simulink model "ClimaCmftFlowMgr"  (R2022b)
settings: Solver=FixedStepDiscrete  StartTime=0.0  StopTime=10.0  FixedStep=1  RelTol=1e-3
settings Model: Creator=blue.ge  LastModifiedBy=blue.ge
settings DataIOCC: SaveFormat=StructureWithTime
settings OptimizationCC: DefaultParameterBehavior=Inlined  EnhancedBackFolding=on  PassReuseOutputArgsAs=Individual arguments  ZeroExternalMemoryAtStartup=off  ZeroInternalMemoryAtStartup=off  OptimizeBlockOrder=speed  DifferentSizesBufferReuse=on  OptimizationCustomize=off  EfficientTunableParamExpr=on
settings DebuggingCC: ParameterTunabilityLossMsg=error
settings ModelReferenceCC: ModelReferenceNumInstancesAllowed=Single
settings SFSimCC: MATLABDynamicMemAlloc=off
settings RTWCC: Description=AUTOSAR  SystemTargetFile=autosar.tlc  GenCodeOnly=on  TemplateMakefile=ert_default_tmf
settings CodeAppCC: ForceParamTrailComments=on  IgnoreCustomStorageClasses=off  OperatorAnnotations=on  SimulinkDataObjDesc=on  SFDataObjDesc=on  InsertBlockDesc=on
settings STFCustomTargetCC: TargetLangStandard=C99 (ISO)  MultiwordLength=256  ParMdlRefBuildCompliant=on  CompOptLevelCompliant=on  IncludeMdlTerminateFcn=off  CombineOutputUpdateFcns=on  SuppressErrorStatus=on  ERTCustomFileBanners=on  SupportNonFinite=off  SupportComplex=off  SupportContinuousTime=off  SupportNonInlinedSFcns=off  RemoveResetFunc=on  MATLABClassNameForMDSCustomization=Simulink.SoftwareTarget.ERTCustomization  AutosarCompliant=on  UseToolchainInfoCompliant=on  LookupTableObjectStructAxisOrder=2,1,3,4,...  LUTObjectStructOrderEvenSpacing=Size,Table,Breakpoints  SystemTargetFile=autosar.tlc
settings ERTTargetCC: TargetLangStandard=C99 (ISO)  ConcurrentExecutionCompliant=off  IncludeMdlTerminateFcn=off  SuppressErrorStatus=on  SupportNonFinite=off  SupportComplex=off  ModelStepFunctionPrototypeControlCompliant=off  CPPClassGenCompliant=off  AutosarCompliant=on  LookupTableObjectStructAxisOrder=2,1,3,4,...  LUTObjectStructOrderEvenSpacing=Size,Table,Breakpoints  InlinedParameterPlacement=NonHierarchical  ERTCustomFileTemplate=example_file_process.tlc

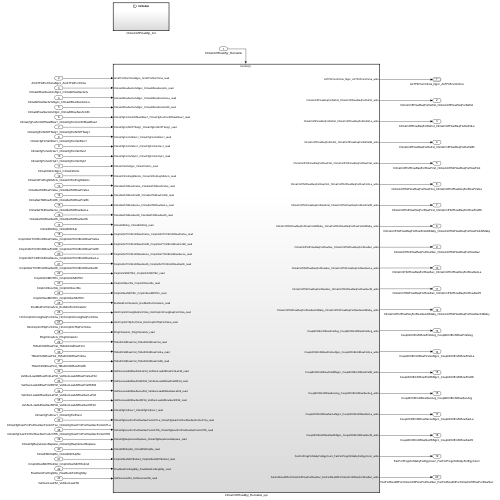
[thumbnail: Simulink's stored preview of the top-level diagram]
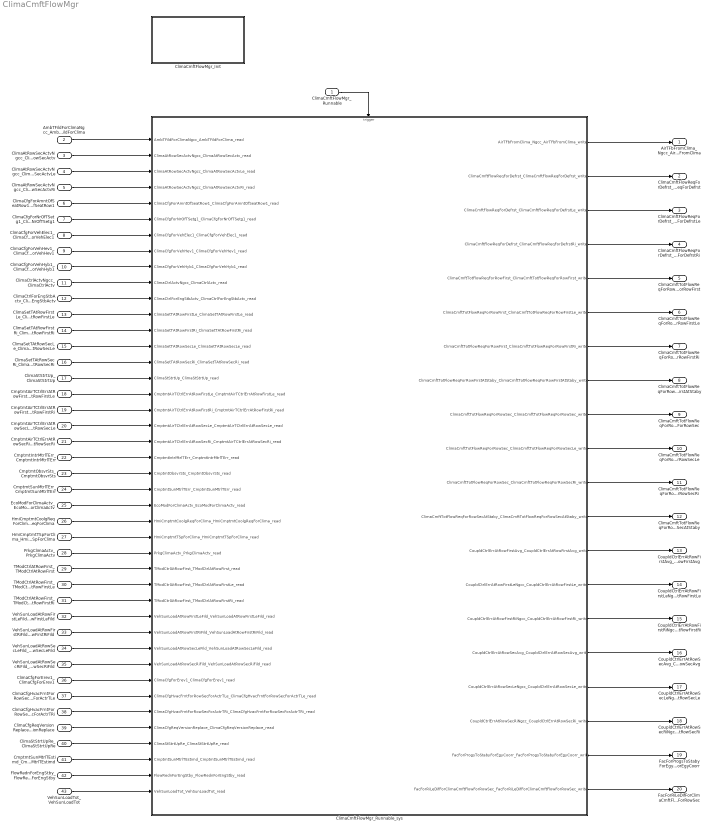
== system ClimaCmftFlowMgr ==
  ClimaCmftFlowMgr_Runnable : Inport  SampleTime=1  OutputFunctionCall=on
  AmbTFildForClimaNgcc_AmbTFildForClima : Inport  Port=2  OutDataTypeStr=T30  PortDimensions=1
  ClimaAtRowSecActvNgcc_ClimaAtRowSecActv : Inport  Port=3  OutDataTypeStr=Boolean  PortDimensions=1
  ClimaAtRowSecActvNgcc_ClimaAtRowSecActvLe : Inport  Port=4  OutDataTypeStr=Boolean  PortDimensions=1
  ClimaAtRowSecActvNgcc_ClimaAtRowSecActvRi : Inport  Port=5  OutDataTypeStr=Boolean  PortDimensions=1
  ClimaCfgForAmntOfSeatRow1_ClimaCfgForAmntOfSeatRow1 : Inport  Port=6  OutDataTypeStr=Enum: ClimaCfgForAmntOfSeatRow1  PortDimensions=1
  ClimaCfgForNrOfTSetg1_ClimaCfgForNrOfTSetg1 : Inport  Port=7  OutDataTypeStr=Enum: ClimaCfgForNrOfTSetg1  PortDimensions=1
  ClimaCfgForVehElec1_ClimaCfgForVehElec1 : Inport  Port=8  OutDataTypeStr=Boolean  PortDimensions=1
  ClimaCfgForVehHev1_ClimaCfgForVehHev1 : Inport  Port=9  OutDataTypeStr=Boolean  PortDimensions=1
  ClimaCfgForVehHyb1_ClimaCfgForVehHyb1 : Inport  Port=10  OutDataTypeStr=Boolean  PortDimensions=1
  ClimaCtrlActvNgcc_ClimaCtrlActv : Inport  Port=11  OutDataTypeStr=Boolean  PortDimensions=1
  ClimaCtrlForEngStbActv_ClimaCtrlForEngStbActv : Inport  Port=12  OutDataTypeStr=Boolean  PortDimensions=1
  ClimaSetTAtRowFirstLe_ClimaSetTAtRowFirstLe : Inport  Port=13  OutDataTypeStr=T30  PortDimensions=1
  ClimaSetTAtRowFirstRi_ClimaSetTAtRowFirstRi : Inport  Port=14  OutDataTypeStr=T30  PortDimensions=1
  ClimaSetTAtRowSecLe_ClimaSetTAtRowSecLe : Inport  Port=15  OutDataTypeStr=T30  PortDimensions=1
  ClimaSetTAtRowSecRi_ClimaSetTAtRowSecRi : Inport  Port=16  OutDataTypeStr=T30  PortDimensions=1
  ClimaStStrtUp_ClimaStStrtUp : Inport  Port=17  OutDataTypeStr=Enum: ClimaStStrtUp  PortDimensions=1
  CmptmtAirTCtrlErrAtRowFirstLe_CmptmtAirTCtrlErrAtRowFirstLe : Inport  Port=18  OutDataTypeStr=T30  PortDimensions=1
  CmptmtAirTCtrlErrAtRowFirstRi_CmptmtAirTCtrlErrAtRowFirstRi : Inport  Port=19  OutDataTypeStr=T30  PortDimensions=1
  CmptmtAirTCtrlErrAtRowSecLe_CmptmtAirTCtrlErrAtRowSecLe : Inport  Port=20  OutDataTypeStr=T30  PortDimensions=1
  CmptmtAirTCtrlErrAtRowSecRi_CmptmtAirTCtrlErrAtRowSecRi : Inport  Port=21  OutDataTypeStr=T30  PortDimensions=1
  CmptmtIntrMtrlTErr_CmptmtIntrMtrlTErr : Inport  Port=22  OutDataTypeStr=T30  PortDimensions=1
  CmptmtObsvrSts_CmptmtObsvrSts : Inport  Port=23  OutDataTypeStr=Enum: ObsvrStsForCmptmt  PortDimensions=1
  CmptmtSunMtrlTErr_CmptmtSunMtrlTErr : Inport  Port=24  OutDataTypeStr=T30  PortDimensions=1
  EcoModForClimaActv_EcoModForClimaActv : Inport  Port=25  OutDataTypeStr=Enum: OnOff1  PortDimensions=1
  HmiCmptmtCoolgReqForClima_HmiCmptmtCoolgReqForClima : Inport  Port=26  OutDataTypeStr=Enum: HmiCmptmtCoolgReq  PortDimensions=1
  HmiCmptmtTSpForClima_HmiCmptmtTSpForClima : Inport  Port=27  OutDataTypeStr=Bus: HmiCmptmtTSp  PortDimensions=1  BusOutputAsStruct=on
  PrkgClimaActv_PrkgClimaActv : Inport  Port=28  OutDataTypeStr=Enum: PrkgClimaActv  PortDimensions=1
  TModCtrlAtRowFirst_TModCtrlAtRowFirst : Inport  Port=29  OutDataTypeStr=T31  PortDimensions=1
  TModCtrlAtRowFirst_TModCtrlAtRowFirstLe : Inport  Port=30  OutDataTypeStr=T31  PortDimensions=1
  TModCtrlAtRowFirst_TModCtrlAtRowFirstRi : Inport  Port=31  OutDataTypeStr=T31  PortDimensions=1
  VehSunLoadAtRowFirstLeFild_VehSunLoadAtRowFirstLeFild : Inport  Port=32  OutDataTypeStr=SunLoad30  PortDimensions=1
  VehSunLoadAtRowFirstRiFild_VehSunLoadAtRowFirstRiFild : Inport  Port=33  OutDataTypeStr=SunLoad30  PortDimensions=1
  VehSunLoadAtRowSecLeFild_VehSunLoadAtRowSecLeFild : Inport  Port=34  OutDataTypeStr=SunLoad30  PortDimensions=1
  VehSunLoadAtRowSecRiFild_VehSunLoadAtRowSecRiFild : Inport  Port=35  OutDataTypeStr=SunLoad30  PortDimensions=1
  ClimaCfgForErev1_ClimaCfgForErev1 : Inport  Port=36  OutDataTypeStr=Boolean  PortDimensions=1
  ClimaCfgHvacFrntForRowSecForActrTLe_ClimaCfgHvacFrntForRowSecForActrTLe : Inport  Port=37  OutDataTypeStr=Boolean  PortDimensions=1
  ClimaCfgHvacFrntForRowSecForActrTRi_ClimaCfgHvacFrntForRowSecForActrTRi : Inport  Port=38  OutDataTypeStr=Boolean  PortDimensions=1
  ClimaCfgReqVersionReplace_ClimaCfgReqVersionReplace : Inport  Port=39  OutDataTypeStr=UInt8  PortDimensions=1
  ClimaStStrtUpRe_ClimaStStrtUpRe : Inport  Port=40  OutDataTypeStr=Enum: ClimaStStrtUp  PortDimensions=1
  CmptmtSunMtrlTEstimd_CmptmtSunMtrlTEstimd : Inport  Port=41  OutDataTypeStr=T30  PortDimensions=1
  FlowRednForEngStby_FlowRednForEngStby : Inport  Port=42  OutDataTypeStr=Boolean  PortDimensions=1
  VehSunLoadTot_VehSunLoadTot : Inport  Port=43  OutDataTypeStr=SunLoad30  PortDimensions=1
  ClimaCmftFlowMgr_Init : Subsystem
  ClimaCmftFlowMgr_Runnable_sys : Subsystem
  AirTFbFromClima_Ngcc_AirTFbFromClima : Outport  OutDataTypeStr=Bus: AirTFbFromClimaRec  PortDimensions=1  BusOutputAsStruct=on
  ClimaCmftFlowReqForDefrst_ClimaCmftFlowReqForDefrst : Outport  Port=2  OutDataTypeStr=AirFlow30  PortDimensions=1
  ClimaCmftFlowReqForDefrst_ClimaCmftFlowReqForDefrstLe : Outport  Port=3  OutDataTypeStr=AirFlow30  PortDimensions=1
  ClimaCmftFlowReqForDefrst_ClimaCmftFlowReqForDefrstRi : Outport  Port=4  OutDataTypeStr=AirFlow30  PortDimensions=1
  ClimaCmftTotFlowReqForRowFirst_ClimaCmftTotFlowReqForRowFirst : Outport  Port=5  OutDataTypeStr=AirFlow30  PortDimensions=1
  ClimaCmftTotFlowReqForRowFirst_ClimaCmftTotFlowReqForRowFirstLe : Outport  Port=6  OutDataTypeStr=AirFlow30  PortDimensions=1
  ClimaCmftTotFlowReqForRowFirst_ClimaCmftTotFlowReqForRowFirstRi : Outport  Port=7  OutDataTypeStr=AirFlow30  PortDimensions=1
  ClimaCmftTotFlowReqForRowFirstAtStaby_ClimaCmftTotFlowReqForRowFirstAtStaby : Outport  Port=8  OutDataTypeStr=AirFlow30  PortDimensions=1
  ClimaCmftTotFlowReqForRowSec_ClimaCmftTotFlowReqForRowSec : Outport  Port=9  OutDataTypeStr=AirFlow30  PortDimensions=1
  ClimaCmftTotFlowReqForRowSec_ClimaCmftTotFlowReqForRowSecLe : Outport  Port=10  OutDataTypeStr=AirFlow30  PortDimensions=1
  ClimaCmftTotFlowReqForRowSec_ClimaCmftTotFlowReqForRowSecRi : Outport  Port=11  OutDataTypeStr=AirFlow30  PortDimensions=1
  ClimaCmftTotFlowReqForRowSecAtStaby_ClimaCmftTotFlowReqForRowSecAtStaby : Outport  Port=12  OutDataTypeStr=AirFlow30  PortDimensions=1
  CoupldCtrlErrAtRowFirstAvg_CoupldCtrlErrAtRowFirstAvg : Outport  Port=13  OutDataTypeStr=T30  PortDimensions=1
  CoupldCtrlErrAtRowFirstLeNgcc_CoupldCtrlErrAtRowFirstLe : Outport  Port=14  OutDataTypeStr=T30  PortDimensions=1
  CoupldCtrlErrAtRowFirstRiNgcc_CoupldCtrlErrAtRowFirstRi : Outport  Port=15  OutDataTypeStr=T30  PortDimensions=1
  CoupldCtrlErrAtRowSecAvg_CoupldCtrlErrAtRowSecAvg : Outport  Port=16  OutDataTypeStr=T30  PortDimensions=1
  CoupldCtrlErrAtRowSecLeNgcc_CoupldCtrlErrAtRowSecLe : Outport  Port=17  OutDataTypeStr=T30  PortDimensions=1
  CoupldCtrlErrAtRowSecRiNgcc_CoupldCtrlErrAtRowSecRi : Outport  Port=18  OutDataTypeStr=T30  PortDimensions=1
  FacForProgsToStabyForEgyCoorr_FacForProgsToStabyForEgyCoorr : Outport  Port=19  OutDataTypeStr=Fac30  PortDimensions=1
  FacForRiLeDifForClimaCmftFlowForRowSec_FacForRiLeDifForClimaCmftFlowForRowSec : Outport  Port=20  OutDataTypeStr=Fac30  PortDimensions=1
  wires:
  AmbTFildForClimaNgcc_AmbTFildForClima/1 -> ClimaCmftFlowMgr_Runnable_sys/1(AmbTFildForClimaNgcc_AmbTFildForClima_read)
  ClimaAtRowSecActvNgcc_ClimaAtRowSecActv/1 -> ClimaCmftFlowMgr_Runnable_sys/2(ClimaAtRowSecActvNgcc_ClimaAtRowSecActv_read)
  ClimaAtRowSecActvNgcc_ClimaAtRowSecActvLe/1 -> ClimaCmftFlowMgr_Runnable_sys/3(ClimaAtRowSecActvNgcc_ClimaAtRowSecActvLe_read)
  ClimaAtRowSecActvNgcc_ClimaAtRowSecActvRi/1 -> ClimaCmftFlowMgr_Runnable_sys/4(ClimaAtRowSecActvNgcc_ClimaAtRowSecActvRi_read)
  ClimaCfgForAmntOfSeatRow1_ClimaCfgForAmntOfSeatRow1/1 -> ClimaCmftFlowMgr_Runnable_sys/5(ClimaCfgForAmntOfSeatRow1_ClimaCfgForAmntOfSeatRow1_read)
  ClimaCfgForNrOfTSetg1_ClimaCfgForNrOfTSetg1/1 -> ClimaCmftFlowMgr_Runnable_sys/6(ClimaCfgForNrOfTSetg1_ClimaCfgForNrOfTSetg1_read)
  ClimaCfgForVehElec1_ClimaCfgForVehElec1/1 -> ClimaCmftFlowMgr_Runnable_sys/7(ClimaCfgForVehElec1_ClimaCfgForVehElec1_read)
  ClimaCfgForVehHev1_ClimaCfgForVehHev1/1 -> ClimaCmftFlowMgr_Runnable_sys/8(ClimaCfgForVehHev1_ClimaCfgForVehHev1_read)
  ClimaCfgForVehHyb1_ClimaCfgForVehHyb1/1 -> ClimaCmftFlowMgr_Runnable_sys/9(ClimaCfgForVehHyb1_ClimaCfgForVehHyb1_read)
  ClimaCtrlActvNgcc_ClimaCtrlActv/1 -> ClimaCmftFlowMgr_Runnable_sys/10(ClimaCtrlActvNgcc_ClimaCtrlActv_read)
  ClimaCtrlForEngStbActv_ClimaCtrlForEngStbActv/1 -> ClimaCmftFlowMgr_Runnable_sys/11(ClimaCtrlForEngStbActv_ClimaCtrlForEngStbActv_read)
  ClimaSetTAtRowFirstLe_ClimaSetTAtRowFirstLe/1 -> ClimaCmftFlowMgr_Runnable_sys/12(ClimaSetTAtRowFirstLe_ClimaSetTAtRowFirstLe_read)
  ClimaSetTAtRowFirstRi_ClimaSetTAtRowFirstRi/1 -> ClimaCmftFlowMgr_Runnable_sys/13(ClimaSetTAtRowFirstRi_ClimaSetTAtRowFirstRi_read)
  ClimaSetTAtRowSecLe_ClimaSetTAtRowSecLe/1 -> ClimaCmftFlowMgr_Runnable_sys/14(ClimaSetTAtRowSecLe_ClimaSetTAtRowSecLe_read)
  ClimaSetTAtRowSecRi_ClimaSetTAtRowSecRi/1 -> ClimaCmftFlowMgr_Runnable_sys/15(ClimaSetTAtRowSecRi_ClimaSetTAtRowSecRi_read)
  ClimaStStrtUp_ClimaStStrtUp/1 -> ClimaCmftFlowMgr_Runnable_sys/16(ClimaStStrtUp_ClimaStStrtUp_read)
  CmptmtAirTCtrlErrAtRowFirstLe_CmptmtAirTCtrlErrAtRowFirstLe/1 -> ClimaCmftFlowMgr_Runnable_sys/17(CmptmtAirTCtrlErrAtRowFirstLe_CmptmtAirTCtrlErrAtRowFirstLe_read)
  CmptmtAirTCtrlErrAtRowFirstRi_CmptmtAirTCtrlErrAtRowFirstRi/1 -> ClimaCmftFlowMgr_Runnable_sys/18(CmptmtAirTCtrlErrAtRowFirstRi_CmptmtAirTCtrlErrAtRowFirstRi_read)
  CmptmtAirTCtrlErrAtRowSecLe_CmptmtAirTCtrlErrAtRowSecLe/1 -> ClimaCmftFlowMgr_Runnable_sys/19(CmptmtAirTCtrlErrAtRowSecLe_CmptmtAirTCtrlErrAtRowSecLe_read)
  CmptmtAirTCtrlErrAtRowSecRi_CmptmtAirTCtrlErrAtRowSecRi/1 -> ClimaCmftFlowMgr_Runnable_sys/20(CmptmtAirTCtrlErrAtRowSecRi_CmptmtAirTCtrlErrAtRowSecRi_read)
  CmptmtIntrMtrlTErr_CmptmtIntrMtrlTErr/1 -> ClimaCmftFlowMgr_Runnable_sys/21(CmptmtIntrMtrlTErr_CmptmtIntrMtrlTErr_read)
  CmptmtObsvrSts_CmptmtObsvrSts/1 -> ClimaCmftFlowMgr_Runnable_sys/22(CmptmtObsvrSts_CmptmtObsvrSts_read)
  CmptmtSunMtrlTErr_CmptmtSunMtrlTErr/1 -> ClimaCmftFlowMgr_Runnable_sys/23(CmptmtSunMtrlTErr_CmptmtSunMtrlTErr_read)
  EcoModForClimaActv_EcoModForClimaActv/1 -> ClimaCmftFlowMgr_Runnable_sys/24(EcoModForClimaActv_EcoModForClimaActv_read)
  HmiCmptmtCoolgReqForClima_HmiCmptmtCoolgReqForClima/1 -> ClimaCmftFlowMgr_Runnable_sys/25(HmiCmptmtCoolgReqForClima_HmiCmptmtCoolgReqForClima_read)
  HmiCmptmtTSpForClima_HmiCmptmtTSpForClima/1 -> ClimaCmftFlowMgr_Runnable_sys/26(HmiCmptmtTSpForClima_HmiCmptmtTSpForClima_read)
  PrkgClimaActv_PrkgClimaActv/1 -> ClimaCmftFlowMgr_Runnable_sys/27(PrkgClimaActv_PrkgClimaActv_read)
  TModCtrlAtRowFirst_TModCtrlAtRowFirst/1 -> ClimaCmftFlowMgr_Runnable_sys/28(TModCtrlAtRowFirst_TModCtrlAtRowFirst_read)
  TModCtrlAtRowFirst_TModCtrlAtRowFirstLe/1 -> ClimaCmftFlowMgr_Runnable_sys/29(TModCtrlAtRowFirst_TModCtrlAtRowFirstLe_read)
  TModCtrlAtRowFirst_TModCtrlAtRowFirstRi/1 -> ClimaCmftFlowMgr_Runnable_sys/30(TModCtrlAtRowFirst_TModCtrlAtRowFirstRi_read)
  VehSunLoadAtRowFirstLeFild_VehSunLoadAtRowFirstLeFild/1 -> ClimaCmftFlowMgr_Runnable_sys/31(VehSunLoadAtRowFirstLeFild_VehSunLoadAtRowFirstLeFild_read)
  VehSunLoadAtRowFirstRiFild_VehSunLoadAtRowFirstRiFild/1 -> ClimaCmftFlowMgr_Runnable_sys/32(VehSunLoadAtRowFirstRiFild_VehSunLoadAtRowFirstRiFild_read)
  VehSunLoadAtRowSecLeFild_VehSunLoadAtRowSecLeFild/1 -> ClimaCmftFlowMgr_Runnable_sys/33(VehSunLoadAtRowSecLeFild_VehSunLoadAtRowSecLeFild_read)
  VehSunLoadAtRowSecRiFild_VehSunLoadAtRowSecRiFild/1 -> ClimaCmftFlowMgr_Runnable_sys/34(VehSunLoadAtRowSecRiFild_VehSunLoadAtRowSecRiFild_read)
  ClimaCfgForErev1_ClimaCfgForErev1/1 -> ClimaCmftFlowMgr_Runnable_sys/35(ClimaCfgForErev1_ClimaCfgForErev1_read)
  ClimaCfgHvacFrntForRowSecForActrTLe_ClimaCfgHvacFrntForRowSecForActrTLe/1 -> ClimaCmftFlowMgr_Runnable_sys/36(ClimaCfgHvacFrntForRowSecForActrTLe_ClimaCfgHvacFrntForRowSecForActrTLe_read)
  ClimaCfgHvacFrntForRowSecForActrTRi_ClimaCfgHvacFrntForRowSecForActrTRi/1 -> ClimaCmftFlowMgr_Runnable_sys/37(ClimaCfgHvacFrntForRowSecForActrTRi_ClimaCfgHvacFrntForRowSecForActrTRi_read)
  ClimaCfgReqVersionReplace_ClimaCfgReqVersionReplace/1 -> ClimaCmftFlowMgr_Runnable_sys/38(ClimaCfgReqVersionReplace_ClimaCfgReqVersionReplace_read)
  ClimaStStrtUpRe_ClimaStStrtUpRe/1 -> ClimaCmftFlowMgr_Runnable_sys/39(ClimaStStrtUpRe_ClimaStStrtUpRe_read)
  CmptmtSunMtrlTEstimd_CmptmtSunMtrlTEstimd/1 -> ClimaCmftFlowMgr_Runnable_sys/40(CmptmtSunMtrlTEstimd_CmptmtSunMtrlTEstimd_read)
  FlowRednForEngStby_FlowRednForEngStby/1 -> ClimaCmftFlowMgr_Runnable_sys/41(FlowRednForEngStby_FlowRednForEngStby_read)
  VehSunLoadTot_VehSunLoadTot/1 -> ClimaCmftFlowMgr_Runnable_sys/42(VehSunLoadTot_VehSunLoadTot_read)
  ClimaCmftFlowMgr_Runnable_sys/1(AirTFbFromClima_Ngcc_AirTFbFromClima_write) -> AirTFbFromClima_Ngcc_AirTFbFromClima/1
  ClimaCmftFlowMgr_Runnable_sys/2(ClimaCmftFlowReqForDefrst_ClimaCmftFlowReqForDefrst_write) -> ClimaCmftFlowReqForDefrst_ClimaCmftFlowReqForDefrst/1
  ClimaCmftFlowMgr_Runnable_sys/3(ClimaCmftFlowReqForDefrst_ClimaCmftFlowReqForDefrstLe_write) -> ClimaCmftFlowReqForDefrst_ClimaCmftFlowReqForDefrstLe/1
  ClimaCmftFlowMgr_Runnable_sys/4(ClimaCmftFlowReqForDefrst_ClimaCmftFlowReqForDefrstRi_write) -> ClimaCmftFlowReqForDefrst_ClimaCmftFlowReqForDefrstRi/1
  ClimaCmftFlowMgr_Runnable_sys/5(ClimaCmftTotFlowReqForRowFirst_ClimaCmftTotFlowReqForRowFirst_write) -> ClimaCmftTotFlowReqForRowFirst_ClimaCmftTotFlowReqForRowFirst/1
  ClimaCmftFlowMgr_Runnable_sys/6(ClimaCmftTotFlowReqForRowFirst_ClimaCmftTotFlowReqForRowFirstLe_write) -> ClimaCmftTotFlowReqForRowFirst_ClimaCmftTotFlowReqForRowFirstLe/1
  ClimaCmftFlowMgr_Runnable_sys/7(ClimaCmftTotFlowReqForRowFirst_ClimaCmftTotFlowReqForRowFirstRi_write) -> ClimaCmftTotFlowReqForRowFirst_ClimaCmftTotFlowReqForRowFirstRi/1
  ClimaCmftFlowMgr_Runnable_sys/8(ClimaCmftTotFlowReqForRowFirstAtStaby_ClimaCmftTotFlowReqForRowFirstAtStaby_write) -> ClimaCmftTotFlowReqForRowFirstAtStaby_ClimaCmftTotFlowReqForRowFirstAtStaby/1
  ClimaCmftFlowMgr_Runnable_sys/9(ClimaCmftTotFlowReqForRowSec_ClimaCmftTotFlowReqForRowSec_write) -> ClimaCmftTotFlowReqForRowSec_ClimaCmftTotFlowReqForRowSec/1
  ClimaCmftFlowMgr_Runnable_sys/10(ClimaCmftTotFlowReqForRowSec_ClimaCmftTotFlowReqForRowSecLe_write) -> ClimaCmftTotFlowReqForRowSec_ClimaCmftTotFlowReqForRowSecLe/1
  ClimaCmftFlowMgr_Runnable_sys/11(ClimaCmftTotFlowReqForRowSec_ClimaCmftTotFlowReqForRowSecRi_write) -> ClimaCmftTotFlowReqForRowSec_ClimaCmftTotFlowReqForRowSecRi/1
  ClimaCmftFlowMgr_Runnable_sys/12(ClimaCmftTotFlowReqForRowSecAtStaby_ClimaCmftTotFlowReqForRowSecAtStaby_write) -> ClimaCmftTotFlowReqForRowSecAtStaby_ClimaCmftTotFlowReqForRowSecAtStaby/1
  ClimaCmftFlowMgr_Runnable_sys/13(CoupldCtrlErrAtRowFirstAvg_CoupldCtrlErrAtRowFirstAvg_write) -> CoupldCtrlErrAtRowFirstAvg_CoupldCtrlErrAtRowFirstAvg/1
  ClimaCmftFlowMgr_Runnable_sys/14(CoupldCtrlErrAtRowFirstLeNgcc_CoupldCtrlErrAtRowFirstLe_write) -> CoupldCtrlErrAtRowFirstLeNgcc_CoupldCtrlErrAtRowFirstLe/1
  ClimaCmftFlowMgr_Runnable_sys/15(CoupldCtrlErrAtRowFirstRiNgcc_CoupldCtrlErrAtRowFirstRi_write) -> CoupldCtrlErrAtRowFirstRiNgcc_CoupldCtrlErrAtRowFirstRi/1
  ClimaCmftFlowMgr_Runnable_sys/16(CoupldCtrlErrAtRowSecAvg_CoupldCtrlErrAtRowSecAvg_write) -> CoupldCtrlErrAtRowSecAvg_CoupldCtrlErrAtRowSecAvg/1
  ClimaCmftFlowMgr_Runnable_sys/17(CoupldCtrlErrAtRowSecLeNgcc_CoupldCtrlErrAtRowSecLe_write) -> CoupldCtrlErrAtRowSecLeNgcc_CoupldCtrlErrAtRowSecLe/1
  ClimaCmftFlowMgr_Runnable_sys/18(CoupldCtrlErrAtRowSecRiNgcc_CoupldCtrlErrAtRowSecRi_write) -> CoupldCtrlErrAtRowSecRiNgcc_CoupldCtrlErrAtRowSecRi/1
  ClimaCmftFlowMgr_Runnable_sys/19(FacForProgsToStabyForEgyCoorr_FacForProgsToStabyForEgyCoorr_write) -> FacForProgsToStabyForEgyCoorr_FacForProgsToStabyForEgyCoorr/1
  ClimaCmftFlowMgr_Runnable_sys/20(FacForRiLeDifForClimaCmftFlowForRowSec_FacForRiLeDifForClimaCmftFlowForRowSec_write) -> FacForRiLeDifForClimaCmftFlowForRowSec_FacForRiLeDifForClimaCmftFlowForRowSec/1
  ClimaCmftFlowMgr_Runnable/1 -> ClimaCmftFlowMgr_Runnable_sys/Trigger

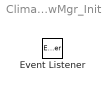
== system ClimaCmftFlowMgr/ClimaCmftFlowMgr_Init ==
  Event Listener : EventListener  EventName=reset

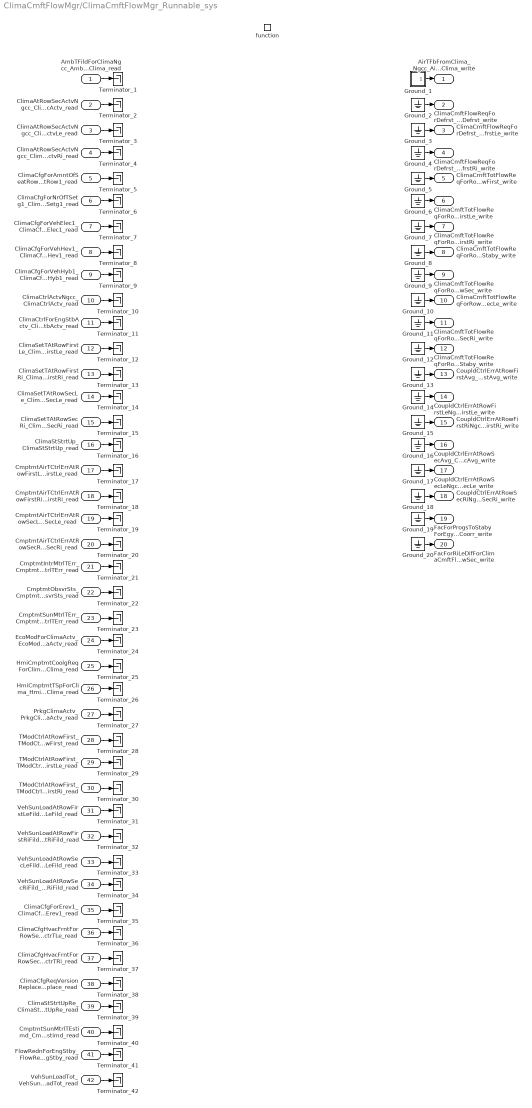
== system ClimaCmftFlowMgr/ClimaCmftFlowMgr_Runnable_sys ==
  AmbTFildForClimaNgcc_AmbTFildForClima_read : Inport
  ClimaAtRowSecActvNgcc_ClimaAtRowSecActv_read : Inport  Port=2
  ClimaAtRowSecActvNgcc_ClimaAtRowSecActvLe_read : Inport  Port=3
  ClimaAtRowSecActvNgcc_ClimaAtRowSecActvRi_read : Inport  Port=4
  ClimaCfgForAmntOfSeatRow1_ClimaCfgForAmntOfSeatRow1_read : Inport  Port=5
  ClimaCfgForNrOfTSetg1_ClimaCfgForNrOfTSetg1_read : Inport  Port=6
  ClimaCfgForVehElec1_ClimaCfgForVehElec1_read : Inport  Port=7
  ClimaCfgForVehHev1_ClimaCfgForVehHev1_read : Inport  Port=8
  ClimaCfgForVehHyb1_ClimaCfgForVehHyb1_read : Inport  Port=9
  ClimaCtrlActvNgcc_ClimaCtrlActv_read : Inport  Port=10
  ClimaCtrlForEngStbActv_ClimaCtrlForEngStbActv_read : Inport  Port=11
  ClimaSetTAtRowFirstLe_ClimaSetTAtRowFirstLe_read : Inport  Port=12
  ClimaSetTAtRowFirstRi_ClimaSetTAtRowFirstRi_read : Inport  Port=13
  ClimaSetTAtRowSecLe_ClimaSetTAtRowSecLe_read : Inport  Port=14
  ClimaSetTAtRowSecRi_ClimaSetTAtRowSecRi_read : Inport  Port=15
  ClimaStStrtUp_ClimaStStrtUp_read : Inport  Port=16
  CmptmtAirTCtrlErrAtRowFirstLe_CmptmtAirTCtrlErrAtRowFirstLe_read : Inport  Port=17
  CmptmtAirTCtrlErrAtRowFirstRi_CmptmtAirTCtrlErrAtRowFirstRi_read : Inport  Port=18
  CmptmtAirTCtrlErrAtRowSecLe_CmptmtAirTCtrlErrAtRowSecLe_read : Inport  Port=19
  CmptmtAirTCtrlErrAtRowSecRi_CmptmtAirTCtrlErrAtRowSecRi_read : Inport  Port=20
  CmptmtIntrMtrlTErr_CmptmtIntrMtrlTErr_read : Inport  Port=21
  CmptmtObsvrSts_CmptmtObsvrSts_read : Inport  Port=22
  CmptmtSunMtrlTErr_CmptmtSunMtrlTErr_read : Inport  Port=23
  EcoModForClimaActv_EcoModForClimaActv_read : Inport  Port=24
  HmiCmptmtCoolgReqForClima_HmiCmptmtCoolgReqForClima_read : Inport  Port=25
  HmiCmptmtTSpForClima_HmiCmptmtTSpForClima_read : Inport  Port=26
  PrkgClimaActv_PrkgClimaActv_read : Inport  Port=27
  TModCtrlAtRowFirst_TModCtrlAtRowFirst_read : Inport  Port=28
  TModCtrlAtRowFirst_TModCtrlAtRowFirstLe_read : Inport  Port=29
  TModCtrlAtRowFirst_TModCtrlAtRowFirstRi_read : Inport  Port=30
  VehSunLoadAtRowFirstLeFild_VehSunLoadAtRowFirstLeFild_read : Inport  Port=31
  VehSunLoadAtRowFirstRiFild_VehSunLoadAtRowFirstRiFild_read : Inport  Port=32
  VehSunLoadAtRowSecLeFild_VehSunLoadAtRowSecLeFild_read : Inport  Port=33
  VehSunLoadAtRowSecRiFild_VehSunLoadAtRowSecRiFild_read : Inport  Port=34
  ClimaCfgForErev1_ClimaCfgForErev1_read : Inport  Port=35
  ClimaCfgHvacFrntForRowSecForActrTLe_ClimaCfgHvacFrntForRowSecForActrTLe_read : Inport  Port=36
  ClimaCfgHvacFrntForRowSecForActrTRi_ClimaCfgHvacFrntForRowSecForActrTRi_read : Inport  Port=37
  ClimaCfgReqVersionReplace_ClimaCfgReqVersionReplace_read : Inport  Port=38
  ClimaStStrtUpRe_ClimaStStrtUpRe_read : Inport  Port=39
  CmptmtSunMtrlTEstimd_CmptmtSunMtrlTEstimd_read : Inport  Port=40
  FlowRednForEngStby_FlowRednForEngStby_read : Inport  Port=41
  VehSunLoadTot_VehSunLoadTot_read : Inport  Port=42
  function : TriggerPort  TriggerType=function-call  VariantControl=(inherit)  SampleTimeType=periodic  SampleTime=-1
  Ground_1 : Subsystem
  Ground_{2..20} (19 alike) : Ground
  Terminator_{1..42} (42 alike) : Terminator
  AirTFbFromClima_Ngcc_AirTFbFromClima_write : Outport
      settings: EnsureOutportIsVirtual=on
  ClimaCmftFlowReqForDefrst_ClimaCmftFlowReqForDefrst_write : Outport  Port=2
      settings: EnsureOutportIsVirtual=on
  ClimaCmftFlowReqForDefrst_ClimaCmftFlowReqForDefrstLe_write : Outport  Port=3
      settings: EnsureOutportIsVirtual=on
  ClimaCmftFlowReqForDefrst_ClimaCmftFlowReqForDefrstRi_write : Outport  Port=4
      settings: EnsureOutportIsVirtual=on
  ClimaCmftTotFlowReqForRowFirst_ClimaCmftTotFlowReqForRowFirst_write : Outport  Port=5
      settings: EnsureOutportIsVirtual=on
  ClimaCmftTotFlowReqForRowFirst_ClimaCmftTotFlowReqForRowFirstLe_write : Outport  Port=6
      settings: EnsureOutportIsVirtual=on
  ClimaCmftTotFlowReqForRowFirst_ClimaCmftTotFlowReqForRowFirstRi_write : Outport  Port=7
      settings: EnsureOutportIsVirtual=on
  ClimaCmftTotFlowReqForRowFirstAtStaby_ClimaCmftTotFlowReqForRowFirstAtStaby_write : Outport  Port=8
      settings: EnsureOutportIsVirtual=on
  ClimaCmftTotFlowReqForRowSec_ClimaCmftTotFlowReqForRowSec_write : Outport  Port=9
      settings: EnsureOutportIsVirtual=on
  ClimaCmftTotFlowReqForRowSec_ClimaCmftTotFlowReqForRowSecLe_write : Outport  Port=10
      settings: EnsureOutportIsVirtual=on
  ClimaCmftTotFlowReqForRowSec_ClimaCmftTotFlowReqForRowSecRi_write : Outport  Port=11
      settings: EnsureOutportIsVirtual=on
  ClimaCmftTotFlowReqForRowSecAtStaby_ClimaCmftTotFlowReqForRowSecAtStaby_write : Outport  Port=12
      settings: EnsureOutportIsVirtual=on
  CoupldCtrlErrAtRowFirstAvg_CoupldCtrlErrAtRowFirstAvg_write : Outport  Port=13
      settings: EnsureOutportIsVirtual=on
  CoupldCtrlErrAtRowFirstLeNgcc_CoupldCtrlErrAtRowFirstLe_write : Outport  Port=14
      settings: EnsureOutportIsVirtual=on
  CoupldCtrlErrAtRowFirstRiNgcc_CoupldCtrlErrAtRowFirstRi_write : Outport  Port=15
      settings: EnsureOutportIsVirtual=on
  CoupldCtrlErrAtRowSecAvg_CoupldCtrlErrAtRowSecAvg_write : Outport  Port=16
      settings: EnsureOutportIsVirtual=on
  CoupldCtrlErrAtRowSecLeNgcc_CoupldCtrlErrAtRowSecLe_write : Outport  Port=17
      settings: EnsureOutportIsVirtual=on
  CoupldCtrlErrAtRowSecRiNgcc_CoupldCtrlErrAtRowSecRi_write : Outport  Port=18
      settings: EnsureOutportIsVirtual=on
  FacForProgsToStabyForEgyCoorr_FacForProgsToStabyForEgyCoorr_write : Outport  Port=19
      settings: EnsureOutportIsVirtual=on
  FacForRiLeDifForClimaCmftFlowForRowSec_FacForRiLeDifForClimaCmftFlowForRowSec_write : Outport  Port=20
      settings: EnsureOutportIsVirtual=on
  wires:
  AmbTFildForClimaNgcc_AmbTFildForClima_read/1 -> Terminator_1/1
  ClimaAtRowSecActvNgcc_ClimaAtRowSecActv_read/1 -> Terminator_2/1
  ClimaAtRowSecActvNgcc_ClimaAtRowSecActvLe_read/1 -> Terminator_3/1
  ClimaAtRowSecActvNgcc_ClimaAtRowSecActvRi_read/1 -> Terminator_4/1
  ClimaCfgForAmntOfSeatRow1_ClimaCfgForAmntOfSeatRow1_read/1 -> Terminator_5/1
  ClimaCfgForNrOfTSetg1_ClimaCfgForNrOfTSetg1_read/1 -> Terminator_6/1
  ClimaCfgForVehElec1_ClimaCfgForVehElec1_read/1 -> Terminator_7/1
  ClimaCfgForVehHev1_ClimaCfgForVehHev1_read/1 -> Terminator_8/1
  ClimaCfgForVehHyb1_ClimaCfgForVehHyb1_read/1 -> Terminator_9/1
  ClimaCtrlActvNgcc_ClimaCtrlActv_read/1 -> Terminator_10/1
  ClimaCtrlForEngStbActv_ClimaCtrlForEngStbActv_read/1 -> Terminator_11/1
  ClimaSetTAtRowFirstLe_ClimaSetTAtRowFirstLe_read/1 -> Terminator_12/1
  ClimaSetTAtRowFirstRi_ClimaSetTAtRowFirstRi_read/1 -> Terminator_13/1
  ClimaSetTAtRowSecLe_ClimaSetTAtRowSecLe_read/1 -> Terminator_14/1
  ClimaSetTAtRowSecRi_ClimaSetTAtRowSecRi_read/1 -> Terminator_15/1
  ClimaStStrtUp_ClimaStStrtUp_read/1 -> Terminator_16/1
  CmptmtAirTCtrlErrAtRowFirstLe_CmptmtAirTCtrlErrAtRowFirstLe_read/1 -> Terminator_17/1
  CmptmtAirTCtrlErrAtRowFirstRi_CmptmtAirTCtrlErrAtRowFirstRi_read/1 -> Terminator_18/1
  CmptmtAirTCtrlErrAtRowSecLe_CmptmtAirTCtrlErrAtRowSecLe_read/1 -> Terminator_19/1
  CmptmtAirTCtrlErrAtRowSecRi_CmptmtAirTCtrlErrAtRowSecRi_read/1 -> Terminator_20/1
  CmptmtIntrMtrlTErr_CmptmtIntrMtrlTErr_read/1 -> Terminator_21/1
  CmptmtObsvrSts_CmptmtObsvrSts_read/1 -> Terminator_22/1
  CmptmtSunMtrlTErr_CmptmtSunMtrlTErr_read/1 -> Terminator_23/1
  EcoModForClimaActv_EcoModForClimaActv_read/1 -> Terminator_24/1
  HmiCmptmtCoolgReqForClima_HmiCmptmtCoolgReqForClima_read/1 -> Terminator_25/1
  HmiCmptmtTSpForClima_HmiCmptmtTSpForClima_read/1 -> Terminator_26/1
  PrkgClimaActv_PrkgClimaActv_read/1 -> Terminator_27/1
  TModCtrlAtRowFirst_TModCtrlAtRowFirst_read/1 -> Terminator_28/1
  TModCtrlAtRowFirst_TModCtrlAtRowFirstLe_read/1 -> Terminator_29/1
  TModCtrlAtRowFirst_TModCtrlAtRowFirstRi_read/1 -> Terminator_30/1
  VehSunLoadAtRowFirstLeFild_VehSunLoadAtRowFirstLeFild_read/1 -> Terminator_31/1
  VehSunLoadAtRowFirstRiFild_VehSunLoadAtRowFirstRiFild_read/1 -> Terminator_32/1
  VehSunLoadAtRowSecLeFild_VehSunLoadAtRowSecLeFild_read/1 -> Terminator_33/1
  VehSunLoadAtRowSecRiFild_VehSunLoadAtRowSecRiFild_read/1 -> Terminator_34/1
  ClimaCfgForErev1_ClimaCfgForErev1_read/1 -> Terminator_35/1
  ClimaCfgHvacFrntForRowSecForActrTLe_ClimaCfgHvacFrntForRowSecForActrTLe_read/1 -> Terminator_36/1
  ClimaCfgHvacFrntForRowSecForActrTRi_ClimaCfgHvacFrntForRowSecForActrTRi_read/1 -> Terminator_37/1
  ClimaCfgReqVersionReplace_ClimaCfgReqVersionReplace_read/1 -> Terminator_38/1
  ClimaStStrtUpRe_ClimaStStrtUpRe_read/1 -> Terminator_39/1
  CmptmtSunMtrlTEstimd_CmptmtSunMtrlTEstimd_read/1 -> Terminator_40/1
  FlowRednForEngStby_FlowRednForEngStby_read/1 -> Terminator_41/1
  VehSunLoadTot_VehSunLoadTot_read/1 -> Terminator_42/1
  Ground_1/1(Out1) -> AirTFbFromClima_Ngcc_AirTFbFromClima_write/1
  Ground_2/1 -> ClimaCmftFlowReqForDefrst_ClimaCmftFlowReqForDefrst_write/1
  Ground_3/1 -> ClimaCmftFlowReqForDefrst_ClimaCmftFlowReqForDefrstLe_write/1
  Ground_4/1 -> ClimaCmftFlowReqForDefrst_ClimaCmftFlowReqForDefrstRi_write/1
  Ground_5/1 -> ClimaCmftTotFlowReqForRowFirst_ClimaCmftTotFlowReqForRowFirst_write/1
  Ground_6/1 -> ClimaCmftTotFlowReqForRowFirst_ClimaCmftTotFlowReqForRowFirstLe_write/1
  Ground_7/1 -> ClimaCmftTotFlowReqForRowFirst_ClimaCmftTotFlowReqForRowFirstRi_write/1
  Ground_8/1 -> ClimaCmftTotFlowReqForRowFirstAtStaby_ClimaCmftTotFlowReqForRowFirstAtStaby_write/1
  Ground_9/1 -> ClimaCmftTotFlowReqForRowSec_ClimaCmftTotFlowReqForRowSec_write/1
  Ground_10/1 -> ClimaCmftTotFlowReqForRowSec_ClimaCmftTotFlowReqForRowSecLe_write/1
  Ground_11/1 -> ClimaCmftTotFlowReqForRowSec_ClimaCmftTotFlowReqForRowSecRi_write/1
  Ground_12/1 -> ClimaCmftTotFlowReqForRowSecAtStaby_ClimaCmftTotFlowReqForRowSecAtStaby_write/1
  Ground_13/1 -> CoupldCtrlErrAtRowFirstAvg_CoupldCtrlErrAtRowFirstAvg_write/1
  Ground_14/1 -> CoupldCtrlErrAtRowFirstLeNgcc_CoupldCtrlErrAtRowFirstLe_write/1
  Ground_15/1 -> CoupldCtrlErrAtRowFirstRiNgcc_CoupldCtrlErrAtRowFirstRi_write/1
  Ground_16/1 -> CoupldCtrlErrAtRowSecAvg_CoupldCtrlErrAtRowSecAvg_write/1
  Ground_17/1 -> CoupldCtrlErrAtRowSecLeNgcc_CoupldCtrlErrAtRowSecLe_write/1
  Ground_18/1 -> CoupldCtrlErrAtRowSecRiNgcc_CoupldCtrlErrAtRowSecRi_write/1
  Ground_19/1 -> FacForProgsToStabyForEgyCoorr_FacForProgsToStabyForEgyCoorr_write/1
  Ground_20/1 -> FacForRiLeDifForClimaCmftFlowForRowSec_FacForRiLeDifForClimaCmftFlowForRowSec_write/1

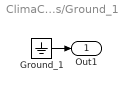
== system ClimaCmftFlowMgr/ClimaCmftFlowMgr_Runnable_sys/Ground_1 ==
  Ground_1 : Ground
  Out1 : Outport  OutDataTypeStr=Bus: AirTFbFromClimaRec  PortDimensions=1
  wires:
  Ground_1/1 -> Out1/1
